simulink library "Renault_Library"  (Simulink 8.2)
settings: Solver=ode3  StartTime=0.0  StopTime=10.0  FixedStep=0.020  RelTol=1e-3
undefined symbols (not defined in the file or in its folder): ABC, AssystemProposal, b, chkval, CoeffA_C, CoeffB_C, CoeffMean_C, ConfTime_C, DiagDelay_C, DividePercent, FailureConfActive_C, FallingEdgeDuration_C, HighLevelThresholdVoltage_C, HighLevelVoltage_C, HighLevelVoltageTunable_C, InitValue_C, InputVoltRangeMax_C, InputVoltRangeMin_C, LowActiveContact_C, LowLevelThresholdVoltage_C, LowLevelVoltage_C, MaxOperatingVoltage_C, MinOperatingVoltage_C, mV2V_conversion, OUT1, OutOfRangeHigh_C, OutOfRangeLow_C, PinSupplySaturationMax, PinSupplySaturationMin, Res1Value_C, Res2Value_C, Res3Value_C, RisingEdgeDuration_C, RSA_CANSignalFailMonitoring, SampleTime_C, service14Table, service19Table, service22Table, service24Table, service28Table, service2ETable, service2FTable, service31Table, service87Table, SizeXStateDID2FTable, SizeXTable14, SizeXTable19, SizeXTable22, SizeXTable24, SizeXTable28, SizeXTable2E, SizeXTable2F, SizeXTable31, SizeXTable87, SizeYStateDID2FTable, SizeYTable14, SizeYTable19, SizeYTable22, SizeYTable24, SizeYTable28 ... (+22)
undefined symbols (not defined in the file): BatteryVoltage_ActivationThreshold_C, FrameStatus_AbsentValue_C, FrameStatus_ConfAbsentValue_C, MemThreshold_C, NAMEOFTHEMODEL_SAMPLE_TIME_CST, NbAbs1_C, NbConfAbs1_C, NbConfPres1_C, NbPres1_C, NOMDUSIGNAL_FaultConfTime_C, NOMDUSIGNAL_FaultRehabTime_C, NOMDUSIGNAL_switchvalue_C, NOMDUSIGNAL_unavailablevalue_C, PeriodFrame1_C, ResetTime_C, WakeUpTime_C
libraries: Renault_Library x112, simulink x75
(part 16 of 32)

== system Renault_Library/Complex blocks/Signal Routing/RSA_SlopeSwitch2Inputs/Slope_On_Block/Output_Comparision ==
  Test_passed : Inport
      settings: AttributesFormatString=Type : %<OutDataTypeStr>
  Input : Inport  Tag=AM,In,0,1,Name,C;CMP,Port,PortWidth,SampleTime;  Port=2
      settings: AttributesFormatString=Type : %<OutDataTypeStr>
  Pos_Slope : Inport  Tag=AM,In,0,1,Name,C;CMP,Port,PortWidth,SampleTime;  Port=3
      settings: AttributesFormatString=Type : %<OutDataTypeStr>
  Prev_Output : Inport  Port=4
      settings: AttributesFormatString=Type : %<OutDataTypeStr>
  Neg_Slope : Inport  Tag=AM,In,0,1,Name,C;CMP,Port,PortWidth,SampleTime;  Port=5
      settings: AttributesFormatString=Type : %<OutDataTypeStr>
  Logical_Operator : Logic  Operator=OR  OutDataTypeStr=boolean
  Logical_Operator2 : Logic  OutDataTypeStr=boolean
  Relational_Operator1 : RelationalOperator  Operator=>  OutDataTypeStr=boolean  InputSameDT=on
  Relational_Operator2 : RelationalOperator  Operator=<  OutDataTypeStr=boolean  InputSameDT=on
  Sum : Sum  OutDataTypeStr=Inherit: Inherit via back propagation  RndMeth=Zero  SaturateOnIntegerOverflow=on  InputSameDT=on
      settings: AttributesFormatString=SAT = %<SaturateOnIntegerOverflow>
  Sum1 : Sum  OutDataTypeStr=Inherit: Inherit via back propagation  RndMeth=Zero  SaturateOnIntegerOverflow=on  InputSameDT=on
      settings: AttributesFormatString=SAT = %<SaturateOnIntegerOverflow>
  Switch1 : Switch  Tag=GEN,Threshold;AM,Switch,2,1;CMP,Threshold;  Criteria=u2 ~= 0  RndMeth=Zero  SaturateOnIntegerOverflow=on  InputSameDT=on
      settings: AttributesFormatString=Criteria=%<Criteria>
  Switch2 : Switch  Tag=GEN,Threshold;AM,Switch,2,1;CMP,Threshold;  Criteria=u2 ~= 0  RndMeth=Zero  SaturateOnIntegerOverflow=on  InputSameDT=on
      settings: AttributesFormatString=Criteria=%<Criteria>
  Switch3 : Switch  Tag=GEN,Threshold;AM,Switch,2,1;CMP,Threshold;  Criteria=u2 ~= 0  RndMeth=Zero  SaturateOnIntegerOverflow=on  InputSameDT=on
      settings: AttributesFormatString=Criteria=%<Criteria>
  Output_Not_Equals_Input : Outport  Tag=AM,Out,1,0,Name,NC;CMP,Port,OutputWhenDisabled,InitialOutput;
      settings: AttributesFormatString=Type : %<OutDataTypeStr>\nInit :%<InitialOutput>
  Output : Outport  Tag=AM,Out,1,0,Name,NC;CMP,Port,OutputWhenDisabled,InitialOutput;  Port=2
      settings: AttributesFormatString=Type : %<OutDataTypeStr>\nInit :%<InitialOutput>
  RelOp2_out : Outport  Tag=AM,Out,1,0,Name,NC;CMP,Port,OutputWhenDisabled,InitialOutput;  Port=3
      settings: AttributesFormatString=Type : %<OutDataTypeStr>\nInit :%<InitialOutput>
  RelOp1_out : Outport  Tag=AM,Out,1,0,Name,NC;CMP,Port,OutputWhenDisabled,InitialOutput;  Port=4
      settings: AttributesFormatString=Type : %<OutDataTypeStr>\nInit :%<InitialOutput>
  wires:
  [RelOp1_out] -> RelOp1_out/1
  [RelOp2_out] -> RelOp2_out/1
  Relational_Operator2/1 -> [RelOp2_out]
  [RelOp1_out] -> Logical_Operator/2
  [Sum_out] -> Switch1/1
  [RelOp2_out] -> Logical_Operator/1
  [RelOp2_out] -> Switch2/2
  [Input] -> Switch3/3
  [Input] -> Switch1/3, Relational_Operator1/2
  [Input] -> Relational_Operator2/2
  Input/1 -> [Input]
  [sw2_out] -> Switch3/1
  Switch2/1 -> [sw2_out]
  [sw1_out] -> Switch2/3
  Switch1/1 -> [sw1_out]
  [Prev_Output] -> Sum/1
  Logical_Operator2/1 -> Output_Not_Equals_Input/1
  Logical_Operator/1 -> Logical_Operator2/2
  Switch3/1 -> Output/1
  Test_passed/1 -> Switch3/2, Logical_Operator2/1
  Relational_Operator1/1 -> [RelOp1_out], Switch1/2
  Sum/1 -> Relational_Operator1/1, [Sum_out]
  Sum1/1 -> Switch2/1, Relational_Operator2/1
  Pos_Slope/1 -> Sum1/2
  Prev_Output/1 -> Sum1/1, [Prev_Output]
  Neg_Slope/1 -> Sum/2

== system Renault_Library/Services ECU/Commonly_Used_Blocks/RSA_ServECU_EnabledSupply/Mean/Moy_1 ==
  In1 : Inport
  CoeffB2 : Constant  OutDataTypeStr=Inherit: Inherit via back propagation  SampleTime=-1
  Divide1 : Product  Inputs=*/  RndMeth=Zero  InputSameDT=on
  Out1 : Outport
  wires:
  CoeffB2/1 -> Divide1/2
  In1/1 -> Divide1/1
  Divide1/1 -> Out1/1

== system Renault_Library/Services ECU/Commonly_Used_Blocks/RSA_ServECU_EnabledSupply/Mean/Moy_10 ==
  In1 : Inport
  CoeffB2 : Constant  Value=10  OutDataTypeStr=Inherit: Inherit via back propagation  SampleTime=-1
  Divide1 : Product  Inputs=*/  RndMeth=Zero  InputSameDT=on
  Output{1..9} (9 alike) : UnitDelay  SampleTime=-1
  SumofElements2 : Sum  Inputs=10  CollapseDim=10  RndMeth=Zero  InputSameDT=on
  Out1 : Outport
  wires:
  SumofElements2/1 -> Divide1/1
  Output8/1 -> SumofElements2/9, Output9/1
  Output9/1 -> SumofElements2/10
  Output7/1 -> SumofElements2/8, Output8/1
  Output6/1 -> SumofElements2/7, Output7/1
  Output5/1 -> SumofElements2/6, Output6/1
  Output4/1 -> SumofElements2/5, Output5/1
  Output3/1 -> SumofElements2/4, Output4/1
  CoeffB2/1 -> Divide1/2
  Output2/1 -> Output3/1, SumofElements2/3
  Output1/1 -> Output2/1, SumofElements2/2
  In1/1 -> Output1/1, SumofElements2/1
  Divide1/1 -> Out1/1

== system Renault_Library/Services ECU/Commonly_Used_Blocks/RSA_ServECU_EnabledSupply/Mean/Moy_2 ==
  In1 : Inport
  CoeffB2 : Constant  Value=2  OutDataTypeStr=Inherit: Inherit via back propagation  SampleTime=-1
  Divide1 : Product  Inputs=*/  RndMeth=Zero  InputSameDT=on
  Output1 : UnitDelay  SampleTime=-1
  SumofElements2 : Sum  Inputs=2  CollapseDim=10  RndMeth=Zero  InputSameDT=on
  Out1 : Outport
  wires:
  SumofElements2/1 -> Divide1/1
  CoeffB2/1 -> Divide1/2
  Output1/1 -> SumofElements2/2
  In1/1 -> Output1/1, SumofElements2/1
  Divide1/1 -> Out1/1

== system Renault_Library/Services ECU/Commonly_Used_Blocks/RSA_ServECU_EnabledSupply/Mean/Moy_3 ==
  In1 : Inport
  CoeffB2 : Constant  Value=3  OutDataTypeStr=Inherit: Inherit via back propagation  SampleTime=-1
  Divide1 : Product  Inputs=*/  RndMeth=Zero  InputSameDT=on
  Output1 : UnitDelay  SampleTime=-1
  Output2 : UnitDelay  SampleTime=-1
  SumofElements1 : Sum  Inputs=2  CollapseDim=10  RndMeth=Zero  InputSameDT=on
  SumofElements2 : Sum  Inputs=2  CollapseDim=10  RndMeth=Zero  InputSameDT=on
  Out1 : Outport
  wires:
  SumofElements2/1 -> Divide1/1
  CoeffB2/1 -> Divide1/2
  Output2/1 -> SumofElements2/2
  Output1/1 -> Output2/1, SumofElements1/2
  In1/1 -> Output1/1, SumofElements1/1
  Divide1/1 -> Out1/1
  SumofElements1/1 -> SumofElements2/1

== system Renault_Library/Services ECU/Commonly_Used_Blocks/RSA_ServECU_EnabledSupply/Mean/Moy_4 ==
  In1 : Inport
  CoeffB2 : Constant  Value=4  OutDataTypeStr=Inherit: Inherit via back propagation  SampleTime=-1
  Divide1 : Product  Inputs=*/  RndMeth=Zero  InputSameDT=on
  Output1 : UnitDelay  SampleTime=-1
  Output2 : UnitDelay  SampleTime=-1
  Output3 : UnitDelay  SampleTime=-1
  SumofElements1 : Sum  Inputs=2  CollapseDim=10  RndMeth=Zero  InputSameDT=on
  SumofElements2 : Sum  Inputs=2  CollapseDim=10  RndMeth=Zero  InputSameDT=on
  SumofElements3 : Sum  Inputs=2  CollapseDim=10  RndMeth=Zero  InputSameDT=on
  Out1 : Outport
  wires:
  SumofElements3/1 -> Divide1/1
  Output3/1 -> SumofElements3/2
  CoeffB2/1 -> Divide1/2
  Output2/1 -> Output3/1, SumofElements2/2
  Output1/1 -> Output2/1, SumofElements1/2
  In1/1 -> Output1/1, SumofElements1/1
  Divide1/1 -> Out1/1
  SumofElements1/1 -> SumofElements2/1
  SumofElements2/1 -> SumofElements3/1

== system Renault_Library/Services ECU/Commonly_Used_Blocks/RSA_ServECU_EnabledSupply/Mean/Moy_5 ==
  In1 : Inport
  CoeffB2 : Constant  Value=5  OutDataTypeStr=Inherit: Inherit via back propagation  SampleTime=-1
  Divide1 : Product  Inputs=*/  RndMeth=Zero  InputSameDT=on
  Output{1..4} (4 alike) : UnitDelay  SampleTime=-1
  SumofElements{1..4} (4 alike) : Sum  Inputs=2  CollapseDim=10  RndMeth=Zero  InputSameDT=on
  Out1 : Outport
  wires:
  Output4/1 -> SumofElements4/2
  Output3/1 -> Output4/1, SumofElements3/2
  CoeffB2/1 -> Divide1/2
  Output2/1 -> Output3/1, SumofElements2/2
  Output1/1 -> Output2/1, SumofElements1/2
  In1/1 -> Output1/1, SumofElements1/1
  Divide1/1 -> Out1/1
  SumofElements3/1 -> SumofElements4/1
  SumofElements1/1 -> SumofElements2/1
  SumofElements2/1 -> SumofElements3/1
  SumofElements4/1 -> Divide1/1

== system Renault_Library/Services ECU/Commonly_Used_Blocks/RSA_ServECU_EnabledSupply/Mean/Moy_6 ==
  In1 : Inport
  CoeffB2 : Constant  Value=6  OutDataTypeStr=Inherit: Inherit via back propagation  SampleTime=-1
  Divide1 : Product  Inputs=*/  RndMeth=Zero  InputSameDT=on
  Output{1..5} (5 alike) : UnitDelay  SampleTime=-1
  SumofElements{1..5} (5 alike) : Sum  Inputs=2  CollapseDim=10  RndMeth=Zero  InputSameDT=on
  Out1 : Outport
  wires:
  SumofElements5/1 -> Divide1/1
  Output5/1 -> SumofElements5/2
  Output4/1 -> Output5/1, SumofElements4/2
  Output3/1 -> Output4/1, SumofElements3/2
  CoeffB2/1 -> Divide1/2
  Output2/1 -> Output3/1, SumofElements2/2
  Output1/1 -> Output2/1, SumofElements1/2
  In1/1 -> Output1/1, SumofElements1/1
  Divide1/1 -> Out1/1
  SumofElements1/1 -> SumofElements2/1
  SumofElements2/1 -> SumofElements3/1
  SumofElements3/1 -> SumofElements4/1
  SumofElements4/1 -> SumofElements5/1

== system Renault_Library/Services ECU/Commonly_Used_Blocks/RSA_ServECU_EnabledSupply/Mean/Moy_7 ==
  In1 : Inport
  CoeffB2 : Constant  Value=7  OutDataTypeStr=Inherit: Inherit via back propagation  SampleTime=-1
  Divide1 : Product  Inputs=*/  RndMeth=Zero  InputSameDT=on
  Output{1..6} (6 alike) : UnitDelay  SampleTime=-1
  SumofElements{1..6} (6 alike) : Sum  Inputs=2  CollapseDim=10  RndMeth=Zero  InputSameDT=on
  Out1 : Outport
  wires:
  SumofElements6/1 -> Divide1/1
  Output6/1 -> SumofElements6/2
  Output5/1 -> Output6/1, SumofElements5/2
  Output4/1 -> Output5/1, SumofElements4/2
  Output3/1 -> Output4/1, SumofElements3/2
  CoeffB2/1 -> Divide1/2
  Output2/1 -> Output3/1, SumofElements2/2
  Output1/1 -> Output2/1, SumofElements1/2
  In1/1 -> Output1/1, SumofElements1/1
  Divide1/1 -> Out1/1
  SumofElements1/1 -> SumofElements2/1
  SumofElements2/1 -> SumofElements3/1
  SumofElements3/1 -> SumofElements4/1
  SumofElements4/1 -> SumofElements5/1
  SumofElements5/1 -> SumofElements6/1

== system Renault_Library/Services ECU/Commonly_Used_Blocks/RSA_ServECU_EnabledSupply/Mean/Moy_8 ==
  In1 : Inport
  CoeffB2 : Constant  Value=8  OutDataTypeStr=Inherit: Inherit via back propagation  SampleTime=-1
  Divide1 : Product  Inputs=*/  RndMeth=Zero  InputSameDT=on
  Output{1..7} (7 alike) : UnitDelay  SampleTime=-1
  SumofElements{1..7} (7 alike) : Sum  Inputs=2  CollapseDim=10  RndMeth=Zero  InputSameDT=on
  Out1 : Outport
  wires:
  Output7/1 -> SumofElements7/2
  Output6/1 -> Output7/1, SumofElements6/2
  Output5/1 -> Output6/1, SumofElements5/2
  Output4/1 -> Output5/1, SumofElements4/2
  Output3/1 -> Output4/1, SumofElements3/2
  CoeffB2/1 -> Divide1/2
  Output2/1 -> Output3/1, SumofElements2/2
  Output1/1 -> Output2/1, SumofElements1/2
  In1/1 -> Output1/1, SumofElements1/1
  Divide1/1 -> Out1/1
  SumofElements7/1 -> Divide1/1
  SumofElements5/1 -> SumofElements6/1
  SumofElements4/1 -> SumofElements5/1
  SumofElements3/1 -> SumofElements4/1
  SumofElements2/1 -> SumofElements3/1
  SumofElements1/1 -> SumofElements2/1
  SumofElements6/1 -> SumofElements7/1

== system Renault_Library/Services ECU/Commonly_Used_Blocks/RSA_ServECU_EnabledSupply/Mean/Moy_9 ==
  In1 : Inport
  CoeffB2 : Constant  Value=9  OutDataTypeStr=Inherit: Inherit via back propagation  SampleTime=-1
  Divide1 : Product  Inputs=*/  RndMeth=Zero  InputSameDT=on
  Output{1..8} (8 alike) : UnitDelay  SampleTime=-1
  SumofElements2 : Sum  Inputs=9  CollapseDim=10  RndMeth=Zero  InputSameDT=on
  Out1 : Outport
  wires:
  SumofElements2/1 -> Divide1/1
  Output8/1 -> SumofElements2/9
  Output7/1 -> SumofElements2/8, Output8/1
  Output6/1 -> SumofElements2/7, Output7/1
  Output5/1 -> SumofElements2/6, Output6/1
  Output4/1 -> SumofElements2/5, Output5/1
  Output3/1 -> SumofElements2/4, Output4/1
  CoeffB2/1 -> Divide1/2
  Output2/1 -> Output3/1, SumofElements2/3
  Output1/1 -> Output2/1, SumofElements2/2
  In1/1 -> Output1/1, SumofElements2/1
  Divide1/1 -> Out1/1

== system Renault_Library/Services ECU/Diag/RSA_DiagnosticManagement/DIAGNOSTIC_SANCTION_TOP/GeneralService ==
  SEG_STATE : Inport
  DIAG_BUFFER_REQUEST : Inport  Port=2
  SESSION_ACTIVE : Inport  Port=3
  REQ_DATA_NUMBER : Inport  Port=4
  General_Server_Response : Stateflow chart  TreatAsAtomicUnit=on
  Server_State : Subsystem
  Service_Supported : Subsystem
  Service_Supported_In_Active_Session : Subsystem
  NEGATIVE_RES_CODE : Outport
  NUMBER_SERVICE : Outport  Port=2  OutDataTypeStr=uint8
  SEND_RES_MESSAGE : Outport  Port=3  OutDataTypeStr=boolean
  REQUEST_RECEIVED : Outport  Port=4  OutDataTypeStr=boolean
  wires:
  General_Server_Response/1(NEGATIVE_RES_CODE) -> NEGATIVE_RES_CODE/1
  SEG_STATE/1 -> Server_State/1(SEG_STATE)
  DIAG_BUFFER_REQUEST/1 -> Service_Supported/1(DIAG_BUFFER_REQUEST), Service_Supported_In_Active_Session/1(DIAG_BUFFER_REQUEST), General_Server_Response/2(DIAG_BUFFER_REQUEST)
  Service_Supported/1(SERVICE_SUPPORTED) -> General_Server_Response/3(SERVICE_SUPPORTED)
  SESSION_ACTIVE/1 -> Service_Supported_In_Active_Session/2(SESSION_ACTIVE)
  Server_State/1(ECU_AVAILABLE) -> General_Server_Response/5(ECU_AVAILABLE)
  Service_Supported_In_Active_Session/1(SERVICE_SUPPORTED_IN_ACTIVE_SESSION) -> General_Server_Response/4(SERVICE_SUPPORTED_IN_ACTIVE_SESSION)
  General_Server_Response/2(NUMBER_SERVICE) -> NUMBER_SERVICE/1
  General_Server_Response/3(SEND_RES_MESSAGE) -> SEND_RES_MESSAGE/1
  General_Server_Response/4(REQUEST_RECEIVED) -> REQUEST_RECEIVED/1
  REQ_DATA_NUMBER/1 -> General_Server_Response/1(REQ_DATA_NUMBER)

== system Renault_Library/Services ECU/Diag/RSA_DiagnosticManagement/DIAGNOSTIC_SANCTION_TOP/Service10 ==
  DIAG_BUFFER_REQUEST : Inport
  REQ_DATA_NUMBER : Inport  Port=2
  Trigger : TriggerPort
  Check_Lenght_Message_Service10 : Subsystem
  Check_Message_Service10 : Stateflow chart  TreatAsAtomicUnit=on
  Constant : Constant  Value=true  OutDataTypeStr=boolean  SampleTime=-1
  SubFunction_Supported_Service10 : Subsystem
  SUPPRESS_POS_RES : Outport
  NEGATIVE_RES_CODE : Outport  Port=2
  SEND_RES_MESSAGE : Outport  Port=3
  wires:
  REQ_DATA_NUMBER/1 -> Check_Lenght_Message_Service10/1(REQ_DATA_NUMBER)
  SubFunction_Supported_Service10/2(SUBFUNCTION_SUPPORTED) -> Check_Message_Service10/1(SUBFUNCTION_SUPPORTED)
  Check_Lenght_Message_Service10/1(LENGTH_MESSAGE_CORRECT) -> Check_Message_Service10/2(LENGTH_MESSAGE_CORRECT)
  DIAG_BUFFER_REQUEST/1 -> SubFunction_Supported_Service10/1(DIAG_BUFFER_REQUEST)
  SubFunction_Supported_Service10/1(SUPPRESS_POS_RES) -> SUPPRESS_POS_RES/1
  Check_Message_Service10/1(NEGATIVE_RES_CODE) -> NEGATIVE_RES_CODE/1
  Constant/1 -> Check_Message_Service10/3(CONDITION_CORRECT)
  Check_Message_Service10/2(SEND_RES_MESSAGE) -> SEND_RES_MESSAGE/1

== system Renault_Library/Services ECU/Diag/RSA_DiagnosticManagement/DIAGNOSTIC_SANCTION_TOP/Service11 ==
  DIAG_BUFFER_REQUEST : Inport
  REQ_DATA_NUMBER : Inport  Port=2
  SESSION_ACTIVE : Inport  Port=3
  Trigger : TriggerPort
  Check_Lenght_Message_Service11 : Subsystem
  Check_Message_Service11 : Stateflow chart  TreatAsAtomicUnit=on
  Constant : Constant  Value=true  OutDataTypeStr=boolean  SampleTime=-1
  Constant1 : Constant  Value=true  OutDataTypeStr=boolean  SampleTime=-1
  SubFunction_In_Active_Session_Service11 : Subsystem
  SubFunction_Supported_Service11 : Subsystem
  SUPPRESS_POS_RES : Outport
  NEGATIVE_RES_CODE : Outport  Port=2
  SEND_RES_MESSAGE : Outport  Port=3
  wires:
  SESSION_ACTIVE/1 -> SubFunction_In_Active_Session_Service11/2(SESSION)
  Check_Message_Service11/1(NEGATIVE_RES_CODE) -> NEGATIVE_RES_CODE/1
  SubFunction_Supported_Service11/1(SUPPRESS_POS_RES) -> SUPPRESS_POS_RES/1
  Check_Lenght_Message_Service11/1(LENGTH_MESSAGE_CORRECT) -> Check_Message_Service11/3(LENGTH_MESSAGE_CORRECT)
  SubFunction_In_Active_Session_Service11/1(SUBFUNCTION_SUPPORTED_IN_ACTIVE_SESSION) -> Check_Message_Service11/2(SUBFUNCTION_SUPPORTED_IN_ACTIVE_SESSION)
  SubFunction_Supported_Service11/2(SUBFUNCTION_SUPPORTED) -> Check_Message_Service11/1(SUBFUNCTION_SUPPORTED)
  REQ_DATA_NUMBER/1 -> Check_Lenght_Message_Service11/1(REQ_DATA_NUMBER)
  DIAG_BUFFER_REQUEST/1 -> SubFunction_In_Active_Session_Service11/1(DIAG_BUFFER_REQUEST), SubFunction_Supported_Service11/1(DIAG_BUFFER_REQUEST)
  Check_Message_Service11/2(SEND_RES_MESSAGE) -> SEND_RES_MESSAGE/1
  Constant/1 -> Check_Message_Service11/4(CONDITION_CORRECT)
  Constant1/1 -> Check_Message_Service11/5(SECURITY_ACCESS_ACCEPTED)

== system Renault_Library/Services ECU/Diag/RSA_DiagnosticManagement/DIAGNOSTIC_SANCTION_TOP/Service14 ==
  REQ_DATA_NUMBER : Inport
  SESSION_ACTIVE : Inport  Port=2
  DIAG_BUFFER_REQUEST : Inport  Port=3
  Trigger : TriggerPort
  Check_Lenght_Message_Service14 : Subsystem
  Check_Message_Service14 : Stateflow chart  TreatAsAtomicUnit=on
  Constant : Constant  Value=true  OutDataTypeStr=boolean  SampleTime=-1
  Parameter_Valid_Service14 : Subsystem
  NEGATIVE_RES_CODE : Outport
  SEND_RES_MESSAGE : Outport  Port=2
  wires:
  REQ_DATA_NUMBER/1 -> Check_Lenght_Message_Service14/1(REQ_DATA_NUMBER)
  Check_Lenght_Message_Service14/1(LENGTH_MESSAGE_CORRECT) -> Check_Message_Service14/1(LENGTH_MESSAGE_CORRECT)
  Check_Message_Service14/1(NEGATIVE_RES_CODE) -> NEGATIVE_RES_CODE/1
  Check_Message_Service14/2(SEND_RES_MESSAGE) -> SEND_RES_MESSAGE/1
  SESSION_ACTIVE/1 -> Parameter_Valid_Service14/2(SESSION_ACTIVE)
  Parameter_Valid_Service14/1(PARAMETER_VALID) -> Check_Message_Service14/3(PARAMETER_VALID)
  DIAG_BUFFER_REQUEST/1 -> Parameter_Valid_Service14/1(DIAG_BUFFER_REQUEST)
  Constant/1 -> Check_Message_Service14/2(CONDITION_CORRECT)

== system Renault_Library/Services ECU/Diag/RSA_DiagnosticManagement/DIAGNOSTIC_SANCTION_TOP/Service19 ==
  REQ_DATA_NUMBER : Inport
  DIAG_BUFFER_REQUEST : Inport  Port=2
  SESSION_ACTIVE : Inport  Port=3
  Trigger : TriggerPort
  Check_Lenght_Message_Service19 : Subsystem
  Check_Message_Service19 : Stateflow chart  TreatAsAtomicUnit=on
  Parameter_Valid_Service19 : Subsystem
  SubFunction_In_Active_Session_Service19 : Subsystem
  SubFunction_Supported_Service19 : Subsystem
  NEGATIVE_RES_CODE : Outport
  SUPPRESS_POS_RES : Outport  Port=2
  SEND_RES_MESSAGE : Outport  Port=3
  wires:
  REQ_DATA_NUMBER/1 -> Check_Lenght_Message_Service19/1(REQ_DATA_NUMBER)
  Check_Lenght_Message_Service19/1(LENGTH_MESSAGE_CORRECT) -> Check_Message_Service19/1(LENGTH_MESSAGE_CORRECT)
  DIAG_BUFFER_REQUEST/1 -> SubFunction_In_Active_Session_Service19/2(DIAG_BUFFER_REQUEST), Parameter_Valid_Service19/1(DIAG_BUFFER_REQUEST), SubFunction_Supported_Service19/1(DIAG_BUFFER_REQUEST)
  SubFunction_Supported_Service19/1(SUBFUNCTION_SUPPORTED) -> Check_Message_Service19/4(SUBFUNCTION_SUPPORTED)
  SubFunction_Supported_Service19/2(SUPPRESS_POS_RES) -> SUPPRESS_POS_RES/1
  SESSION_ACTIVE/1 -> SubFunction_In_Active_Session_Service19/1(SESSION)
  SubFunction_In_Active_Session_Service19/1(SUBFUNCTION_SUPPORTED_IN_ACTIVE_SESSION) -> Check_Message_Service19/2(SUBFUNCTION_SUPPORTED_IN_ACTIVE_SESSION)
  Parameter_Valid_Service19/1(PARAMETER_VALID) -> Check_Message_Service19/3(PARAMETER_VALID)
  Check_Message_Service19/1(NEGATIVE_RES_CODE) -> NEGATIVE_RES_CODE/1
  Check_Message_Service19/2(SEND_RES_MESSAGE) -> SEND_RES_MESSAGE/1

== system Renault_Library/Services ECU/Diag/RSA_DiagnosticManagement/DIAGNOSTIC_SANCTION_TOP/Service22 ==
  REQ_DATA_NUMBER : Inport
  DIAG_BUFFER_REQUEST : Inport  Port=2
  SESSION_ACTIVE : Inport  Port=3
  Trigger : TriggerPort
  Check_Lenght_Message_Response_Service22 : Subsystem
  Check_Lenght_Message_Service22 : Subsystem
  Check_Message_Service22 : Stateflow chart  TreatAsAtomicUnit=on
  Constant : Constant  Value=true  OutDataTypeStr=boolean  SampleTime=-1
  Parameter_Valid_Service22 : Subsystem
  NEGATIVE_RES_CODE : Outport
  LIST_DID_SERVICE_22 : Outport  Port=2  PortDimensions=[2046 3]
  SEND_RES_MESSAGE : Outport  Port=3
  wires:
  Check_Lenght_Message_Service22/1(LENGTH_MESSAGE_CORRECT) -> Check_Message_Service22/1(LENGTH_MESSAGE_CORRECT)
  REQ_DATA_NUMBER/1 -> Check_Lenght_Message_Service22/1(REQ_DATA_NUMBER), Parameter_Valid_Service22/1(REQ_DATA_NUMBER)
  Check_Message_Service22/1(NEGATIVE_RES_CODE) -> NEGATIVE_RES_CODE/1
  DIAG_BUFFER_REQUEST/1 -> Parameter_Valid_Service22/2(DIAG_BUFFER_REQUEST)
  Parameter_Valid_Service22/3(PARAMETER_VALID) -> Check_Message_Service22/5(PARAMETER_VALID)
  Parameter_Valid_Service22/4(LIST_DID_SERVICE_22) -> LIST_DID_SERVICE_22/1
  Constant/1 -> Check_Message_Service22/2(CONDITION_CORRECT)
  Check_Lenght_Message_Response_Service22/1(LENGTH_RESPONSE_CORRECT) -> Check_Message_Service22/3(LENGTH_RESPONSE_CORRECT)
  Parameter_Valid_Service22/1(RESPONSE_SIZE) -> Check_Lenght_Message_Response_Service22/1(RESPONSE_SIZE)
  Parameter_Valid_Service22/2(SECURITY_ACCESS_ACCEPTED) -> Check_Message_Service22/4(SECURITY_ACCESS_ACCEPTED)
  SESSION_ACTIVE/1 -> Parameter_Valid_Service22/3(SESSION_ACTIVE)
  Check_Message_Service22/2(SEND_RES_MESSAGE) -> SEND_RES_MESSAGE/1

== system Renault_Library/Services ECU/Diag/RSA_DiagnosticManagement/DIAGNOSTIC_SANCTION_TOP/Service23 ==
  REQ_DATA_NUMBER : Inport
  DIAG_BUFFER_REQUEST : Inport  Port=2
  SESSION_ACTIVE : Inport  Port=3
  Trigger : TriggerPort
  Check_Lenght_Message_Service23 : Subsystem
  Check_Message_Service23 : Stateflow chart  TreatAsAtomicUnit=on
  Constant : Constant  Value=true  OutDataTypeStr=boolean  SampleTime=-1
  Parameter_Valid_Service23 : Subsystem
  NEGATIVE_RES_CODE : Outport
  SEND_RES_MESSAGE : Outport  Port=2
  wires:
  Check_Lenght_Message_Service23/1(LENGTH_MESSAGE_CORRECT) -> Check_Message_Service23/1(LENGTH_MESSAGE_CORRECT)
  REQ_DATA_NUMBER/1 -> Check_Lenght_Message_Service23/1(REQ_DATA_NUMBER), Parameter_Valid_Service23/1(REQ_DATA_NUMBER)
  Check_Message_Service23/1(NEGATIVE_RES_CODE) -> NEGATIVE_RES_CODE/1
  DIAG_BUFFER_REQUEST/1 -> Parameter_Valid_Service23/2(DIAG_BUFFER_REQUEST)
  Constant/1 -> Check_Message_Service23/2(CONDITION_CORRECT)
  SESSION_ACTIVE/1 -> Parameter_Valid_Service23/3(SESSION_ACTIVE)
  Check_Message_Service23/2(SEND_RES_MESSAGE) -> SEND_RES_MESSAGE/1
  Parameter_Valid_Service23/1(SECURITY_ACCESS_ACCEPTED) -> Check_Message_Service23/3(SECURITY_ACCESS_ACCEPTED)
  Parameter_Valid_Service23/2(PARAMETER_VALID) -> Check_Message_Service23/4(PARAMETER_VALID)

== system Renault_Library/Services ECU/Diag/RSA_DiagnosticManagement/DIAGNOSTIC_SANCTION_TOP/Service24 ==
  REQ_DATA_NUMBER : Inport
  DIAG_BUFFER_REQUEST : Inport  Port=2
  SESSION_ACTIVE : Inport  Port=3
  Trigger : TriggerPort
  Check_Lenght_Message_Service24 : Subsystem
  Check_Message_Service24 : Stateflow chart  TreatAsAtomicUnit=on
  Constant : Constant  Value=true  OutDataTypeStr=boolean  SampleTime=-1
  Parameter_Valid_Service24 : Subsystem
  NEGATIVE_RES_CODE : Outport
  SEND_RES_MESSAGE : Outport  Port=2
  wires:
  Check_Lenght_Message_Service24/1(LENGTH_MESSAGE_CORRECT) -> Check_Message_Service24/1(LENGTH_MESSAGE_CORRECT)
  REQ_DATA_NUMBER/1 -> Check_Lenght_Message_Service24/1(REQ_DATA_NUMBER)
  Check_Message_Service24/1(NEGATIVE_RES_CODE) -> NEGATIVE_RES_CODE/1
  DIAG_BUFFER_REQUEST/1 -> Parameter_Valid_Service24/1(DIAG_BUFFER_REQUEST)
  Parameter_Valid_Service24/2(PARAMETER_VALID) -> Check_Message_Service24/4(PARAMETER_VALID)
  Constant/1 -> Check_Message_Service24/2(CONDITION_CORRECT)
  Parameter_Valid_Service24/1(SECURITY_ACCESS_ACCEPTED) -> Check_Message_Service24/3(SECURITY_ACCESS_ACCEPTED)
  SESSION_ACTIVE/1 -> Parameter_Valid_Service24/2(SESSION_ACTIVE)
  Check_Message_Service24/2(SEND_RES_MESSAGE) -> SEND_RES_MESSAGE/1

== system Renault_Library/Services ECU/Diag/RSA_DiagnosticManagement/DIAGNOSTIC_SANCTION_TOP/Service27 ==
  REQ_DATA_NUMBER : Inport
  DIAG_BUFFER_REQUEST : Inport  Port=2
  SESSION_ACTIVE : Inport  Port=3
  Trigger : TriggerPort
  Check_Lenght_Message_Service27 : Subsystem
  Check_Message_Service27 : Stateflow chart  TreatAsAtomicUnit=on
  Constant{,1..3} (4 alike) : Constant  Value=true  OutDataTypeStr=boolean  SampleTime=-1
  SubFunction_In_Active_Session_Service27 : Subsystem
  SubFunction_Supported_Service27 : Subsystem
  NEGATIVE_RES_CODE : Outport
  SUPPRESS_POS_RES : Outport  Port=2
  SEND_RES_MESSAGE : Outport  Port=3
  wires:
  REQ_DATA_NUMBER/1 -> Check_Lenght_Message_Service27/1(REQ_DATA_NUMBER)
  Check_Lenght_Message_Service27/1(LENGTH_MESSAGE_CORRECT) -> Check_Message_Service27/1(LENGTH_MESSAGE_CORRECT)
  DIAG_BUFFER_REQUEST/1 -> SubFunction_Supported_Service27/1(DIAG_BUFFER_REQUEST), SubFunction_In_Active_Session_Service27/2(DIAG_BUFFER_REQUEST)
  SubFunction_Supported_Service27/1(SUBFUNCTION_SUPPORTED) -> Check_Message_Service27/7(SUBFUNCTION_SUPPORTED)
  SubFunction_Supported_Service27/2(SUPPRESS_POS_RES) -> SUPPRESS_POS_RES/1
  Check_Message_Service27/1(NEGATIVE_RES_CODE) -> NEGATIVE_RES_CODE/1
  SESSION_ACTIVE/1 -> SubFunction_In_Active_Session_Service27/1(SESSION)
  SubFunction_In_Active_Session_Service27/1(SUBFUNCTION_SUPPORTED_IN_ACTIVE_SESSION) -> Check_Message_Service27/6(SUBFUNCTION_SUPPORTED_IN_ACTIVE_SESSION)
  Check_Message_Service27/2(SEND_RES_MESSAGE) -> SEND_RES_MESSAGE/1
  Constant/1 -> Check_Message_Service27/2(CONDITION_CORRECT)
  Constant1/1 -> Check_Message_Service27/3(REQ_SEQUENCE_OK)
  Constant2/1 -> Check_Message_Service27/4(KEY_VALID)
  Constant3/1 -> Check_Message_Service27/5(PARAMETER_VALID)

== system Renault_Library/Services ECU/Diag/RSA_DiagnosticManagement/DIAGNOSTIC_SANCTION_TOP/Service28 ==
  REQ_DATA_NUMBER : Inport
  DIAG_BUFFER_REQUEST : Inport  Port=2
  SESSION_ACTIVE : Inport  Port=3
  Trigger : TriggerPort
  Check_Lenght_Message_Service28 : Subsystem
  Check_Message_Service28 : Stateflow chart  TreatAsAtomicUnit=on
  Constant : Constant  Value=true  OutDataTypeStr=boolean  SampleTime=-1
  Parameter_Valid_Service28 : Subsystem
  SubFunction_In_Active_Session_Service28 : Subsystem
  SubFunction_Supported_Service28 : Subsystem
  NEGATIVE_RES_CODE : Outport
  SUPPRESS_POS_RES : Outport  Port=2
  SEND_RES_MESSAGE : Outport  Port=3
  wires:
  REQ_DATA_NUMBER/1 -> Check_Lenght_Message_Service28/1(REQ_DATA_NUMBER)
  DIAG_BUFFER_REQUEST/1 -> SubFunction_Supported_Service28/1(DIAG_BUFFER_OUTPUT), SubFunction_In_Active_Session_Service28/2(DIAG_BUFFER_REQUEST), Parameter_Valid_Service28/1(DIAG_BUFFER_REQUEST)
  SubFunction_Supported_Service28/1(SUBFUNCTION_SUPPORTED) -> Check_Message_Service28/5(SUBFUNCTION_SUPPORTED)
  Check_Message_Service28/1(NEGATIVE_RES_CODE) -> NEGATIVE_RES_CODE/1
  SubFunction_Supported_Service28/2(SUPPRESS_POS_RES) -> SUPPRESS_POS_RES/1
  SubFunction_In_Active_Session_Service28/1(SUBFUNCTION_SUPPORTED_IN_ACTIVE_SESSION) -> Check_Message_Service28/3(SUBFUNCTION_SUPPORTED_IN_ACTIVE_SESSION)
  SESSION_ACTIVE/1 -> SubFunction_In_Active_Session_Service28/1(SESSION)
  Check_Lenght_Message_Service28/1(LENGTH_MESSAGE_CORRECT) -> Check_Message_Service28/1(LENGTH_MESSAGE_CORRECT)
  Check_Message_Service28/2(SEND_RES_MESSAGE) -> SEND_RES_MESSAGE/1
  Parameter_Valid_Service28/1(PARAMETER_VALID) -> Check_Message_Service28/4(PARAMETER_VALID)
  Constant/1 -> Check_Message_Service28/2(CONDITION_CORRECT)

== system Renault_Library/Services ECU/Diag/RSA_DiagnosticManagement/DIAGNOSTIC_SANCTION_TOP/Service2E ==
  REQ_DATA_NUMBER : Inport
  DIAG_BUFFER_REQUEST : Inport  Port=2
  SESSION_ACTIVE : Inport  Port=3
  Trigger : TriggerPort
  Check_Lenght_Message_Service2E : Subsystem
  Check_Message_Service2E : Stateflow chart  TreatAsAtomicUnit=on
  Constant : Constant  Value=true  OutDataTypeStr=boolean  SampleTime=-1
  Constant1 : Constant  Value=true  OutDataTypeStr=boolean  SampleTime=-1
  Parameter_Valid_Service2E : Subsystem
  NEGATIVE_RES_CODE : Outport
  SEND_RES_MESSAGE : Outport  Port=2
  wires:
  REQ_DATA_NUMBER/1 -> Check_Lenght_Message_Service2E/1(REQ_DATA_NUMBER), Parameter_Valid_Service2E/1(REQ_DATA_NUMBER)
  Check_Lenght_Message_Service2E/1(LENGTH_MESSAGE_CORRECT) -> Check_Message_Service2E/1(LENGTH_MESSAGE_CORRECT)
  Constant/1 -> Check_Message_Service2E/2(CONDITION_CORRECT)
  Constant1/1 -> Check_Message_Service2E/3(CORRECTLY_ALTERED_INTO_MEMORY)
  DIAG_BUFFER_REQUEST/1 -> Parameter_Valid_Service2E/3(DIAG_BUFFER_REQUEST)
  SESSION_ACTIVE/1 -> Parameter_Valid_Service2E/2(SESSION_ACTIVE)
  Parameter_Valid_Service2E/2(PARAMETER_VALID) -> Check_Message_Service2E/5(PARAMETER_VALID)
  Parameter_Valid_Service2E/1(SECURITY_ACCESS_ACCEPTED) -> Check_Message_Service2E/4(SECURITY_ACCESS_ACCEPTED)
  Check_Message_Service2E/2(SEND_RES_MESSAGE) -> SEND_RES_MESSAGE/1
  Check_Message_Service2E/1(NEGATIVE_RES_CODE) -> NEGATIVE_RES_CODE/1

== system Renault_Library/Services ECU/Diag/RSA_DiagnosticManagement/DIAGNOSTIC_SANCTION_TOP/Service2F ==
  REQ_DATA_NUMBER : Inport
  SESSION_ACTIVE : Inport  Port=2
  DIAG_BUFFER_REQUEST : Inport  Port=3
  Trigger : TriggerPort
  Check_Lenght_Message_Service2F : Subsystem
  Check_Message_Service2F : Stateflow chart  TreatAsAtomicUnit=on
  Constant : Constant  Value=true  OutDataTypeStr=boolean  SampleTime=-1
  Parameter_Valid_Service2F : Subsystem
  NEGATIVE_RES_CODE : Outport
  SEND_RES_MESSAGE : Outport  Port=2
  wires:
  REQ_DATA_NUMBER/1 -> Check_Lenght_Message_Service2F/1(REQ_DATA_NUMBER)
  Check_Lenght_Message_Service2F/1(LENGTH_MESSAGE_CORRECT) -> Check_Message_Service2F/1(LENGTH_MESSAGE_CORRECT)
  Check_Message_Service2F/1(NEGATIVE_RES_CODE) -> NEGATIVE_RES_CODE/1
  Check_Message_Service2F/2(SEND_RES_MESSAGE) -> SEND_RES_MESSAGE/1
  Parameter_Valid_Service2F/2(PARAMETER_VALID) -> Check_Message_Service2F/4(PARAMETER_VALID)
  SESSION_ACTIVE/1 -> Parameter_Valid_Service2F/1(SESSION_ACTIVE)
  DIAG_BUFFER_REQUEST/1 -> Parameter_Valid_Service2F/2(DIAG_BUFFER_REQUEST)
  Parameter_Valid_Service2F/1(SECURITY_ACCESS_ACCEPTED) -> Check_Message_Service2F/3(SECURITY_ACCESS_ACCEPTED)
  Constant/1 -> Check_Message_Service2F/2(CONDITION_CORRECT)

== system Renault_Library/Services ECU/Diag/RSA_DiagnosticManagement/DIAGNOSTIC_SANCTION_TOP/Service31 ==
  REQ_DATA_NUMBER : Inport
  DIAG_BUFFER_REQUEST : Inport  Port=2
  SESSION_ACTIVE : Inport  Port=3
  Trigger : TriggerPort
  Check_Lenght_Message_Service31 : Subsystem
  Check_Message_Service31 : Stateflow chart  TreatAsAtomicUnit=on
  Constant : Constant  Value=true  OutDataTypeStr=boolean  SampleTime=-1
  Constant1 : Constant  Value=true  OutDataTypeStr=boolean  SampleTime=-1
  Parameter_Valid_Service31 : Subsystem
  SubFunction_In_Active_Session_Service31 : Subsystem
  SubFunction_Supported_Service31 : Subsystem
  NEGATIVE_RES_CODE : Outport
  SUPPRESS_POS_RES : Outport  Port=2
  SEND_RES_MESSAGE : Outport  Port=3
  wires:
  REQ_DATA_NUMBER/1 -> Check_Lenght_Message_Service31/1(REQ_DATA_NUMBER)
  Check_Lenght_Message_Service31/1(LENGTH_MESSAGE_CORRECT) -> Check_Message_Service31/1(LENGTH_MESSAGE_CORRECT)
  Check_Message_Service31/1(NEGATIVE_RES_CODE) -> NEGATIVE_RES_CODE/1
  SubFunction_Supported_Service31/1(SUBFUNCTION_SUPPORTED) -> Check_Message_Service31/7(SUBFUNCTION_SUPPORTED)
  DIAG_BUFFER_REQUEST/1 -> SubFunction_In_Active_Session_Service31/2(DIAG_BUFFER_REQUEST), SubFunction_Supported_Service31/1(DIAG_BUFFER_REQUEST), [DIAG_BUFFER_REQUEST]
  SubFunction_Supported_Service31/2(SUPPRESS_POS_RES) -> SUPPRESS_POS_RES/1
  SubFunction_In_Active_Session_Service31/1(SUBFUNCTION_SUPPORTED_IN_ACTIVE_SESSION) -> Check_Message_Service31/6(SUBFUNCTION_SUPPORTED_IN_ACTIVE_SESSION)
  SESSION_ACTIVE/1 -> SubFunction_In_Active_Session_Service31/1(SESSION), Parameter_Valid_Service31/2(SESSION_ACTIVE)
  Check_Message_Service31/2(SEND_RES_MESSAGE) -> SEND_RES_MESSAGE/1
  [DIAG_BUFFER_REQUEST] -> Parameter_Valid_Service31/1(DIAG_BUFFER_REQUEST)
  Parameter_Valid_Service31/1(SECURITY_ACCESS_ACCEPTED) -> Check_Message_Service31/4(SECURITY_ACCESS_ACCEPTED)
  Parameter_Valid_Service31/2(PARAMETER_VALID) -> Check_Message_Service31/5(PARAMETER_VALID)
  Constant/1 -> Check_Message_Service31/2(REQ_SEQUENCE_OK)
  Constant1/1 -> Check_Message_Service31/3(CONDITION_CORRECT)

== system Renault_Library/Services ECU/Diag/RSA_DiagnosticManagement/DIAGNOSTIC_SANCTION_TOP/Service3D ==
  REQ_DATA_NUMBER : Inport
  DIAG_BUFFER_REQUEST : Inport  Port=2
  SESSION_ACTIVE : Inport  Port=3
  Trigger : TriggerPort
  Check_Lenght_Message_Service3D : Subsystem
  Check_Message_Service3D : Stateflow chart  TreatAsAtomicUnit=on
  Constant : Constant  Value=true  OutDataTypeStr=boolean  SampleTime=-1
  Constant1 : Constant  Value=true  OutDataTypeStr=boolean  SampleTime=-1
  Parameter_Valid_Service3D : Subsystem
  NEGATIVE_RES_CODE : Outport
  SEND_RES_MESSAGE : Outport  Port=2
  wires:
  Check_Message_Service3D/1(NEGATIVE_RES_CODE) -> NEGATIVE_RES_CODE/1
  Check_Message_Service3D/2(SEND_RES_MESSAGE) -> SEND_RES_MESSAGE/1
  SESSION_ACTIVE/1 -> Parameter_Valid_Service3D/2(SESSION_ACTIVE)
  DIAG_BUFFER_REQUEST/1 -> Parameter_Valid_Service3D/3(DIAG_BUFFER_REQUEST)
  Constant1/1 -> Check_Message_Service3D/3(CORRECTLY_ALTERED_INTO_MEMORY)
  Constant/1 -> Check_Message_Service3D/2(CONDITION_CORRECT)
  Check_Lenght_Message_Service3D/1(LENGTH_MESSAGE_CORRECT) -> Check_Message_Service3D/1(LENGTH_MESSAGE_CORRECT)
  REQ_DATA_NUMBER/1 -> Parameter_Valid_Service3D/1(REQ_DATA_NUMBER), Check_Lenght_Message_Service3D/1(REQ_DATA_NUMBER)
  Parameter_Valid_Service3D/1(SECURITY_ACCESS_ACCEPTED) -> Check_Message_Service3D/4(SECURITY_ACCESS_ACCEPTED)
  Parameter_Valid_Service3D/2(PARAMETER_VALID) -> Check_Message_Service3D/5(PARAMETER_VALID)

== system Renault_Library/Services ECU/Diag/RSA_DiagnosticManagement/DIAGNOSTIC_SANCTION_TOP/Service3E ==
  REQ_DATA_NUMBER : Inport
  DIAG_BUFFER_REQUEST : Inport  Port=2
  SESSION_ACTIVE : Inport  Port=3
  Trigger : TriggerPort
  Check_Lenght_Message_Service3E : Subsystem
  Check_Message_Service3E : Stateflow chart  TreatAsAtomicUnit=on
  SubFunction_In_Active_Session_Service3E : Subsystem
  SubFunction_Supported_Service3E : Subsystem
  SUPPRESS_POS_RES : Outport
  NEGATIVE_RES_CODE : Outport  Port=2
  SEND_RES_MESSAGE : Outport  Port=3
  wires:
  SubFunction_Supported_Service3E/1(SUBFUNCTION_SUPPORTED) -> Check_Message_Service3E/3(SUBFUNCTION_SUPPORTED)
  REQ_DATA_NUMBER/1 -> Check_Lenght_Message_Service3E/1(REQ_DATA_NUMBER)
  Check_Lenght_Message_Service3E/1(LENGTH_MESSAGE_CORRECT) -> Check_Message_Service3E/1(LENGTH_MESSAGE_CORRECT)
  SubFunction_Supported_Service3E/2(SUPPRESS_POS_RES) -> SUPPRESS_POS_RES/1
  SESSION_ACTIVE/1 -> SubFunction_In_Active_Session_Service3E/1(SESSION)
  SubFunction_In_Active_Session_Service3E/1(SUBFUNCTION_SUPPORTED_IN_ACTIVE_SESSION) -> Check_Message_Service3E/2(SUBFUNCTION_SUPPORTED_IN_ACTIVE_SESSION)
  DIAG_BUFFER_REQUEST/1 -> SubFunction_In_Active_Session_Service3E/2(DIAG_BUFFER_REQUEST), SubFunction_Supported_Service3E/1(DIAG_BUFFER_REQUEST)
  Check_Message_Service3E/2(SEND_RES_MESSAGE) -> SEND_RES_MESSAGE/1
  Check_Message_Service3E/1(NEGATIVE_RES_CODE) -> NEGATIVE_RES_CODE/1

== system Renault_Library/Services ECU/Diag/RSA_DiagnosticManagement/DIAGNOSTIC_SANCTION_TOP/Service87 ==
  REQ_DATA_NUMBER : Inport
  DIAG_BUFFER_REQUEST : Inport  Port=2
  SESSION_ACTIVE : Inport  Port=3
  Trigger : TriggerPort
  Check_Lenght_Message_Service87 : Subsystem
  Check_Message_Service87 : Stateflow chart  TreatAsAtomicUnit=on
  Constant : Constant  Value=true  OutDataTypeStr=boolean  SampleTime=-1
  Constant1 : Constant  Value=true  OutDataTypeStr=boolean  SampleTime=-1
  Parameter_Valid_Service87 : Subsystem
  SubFunction_In_Active_Session_Service87 : Subsystem
  SubFunction_Supported_Service87 : Subsystem
  NEGATIVE_RES_CODE : Outport
  SUPPRESS_POS_RES : Outport  Port=2
  SEND_RES_MESSAGE : Outport  Port=3
  wires:
  Parameter_Valid_Service87/1(PARAMETER_VALID) -> Check_Message_Service87/5(PARAMETER_VALID)
  Constant1/1 -> Check_Message_Service87/3(REQ_SEQUENCE_OK)
  Constant/1 -> Check_Message_Service87/2(CONDITION_CORRECT)
  Check_Message_Service87/2(SEND_RES_MESSAGE) -> SEND_RES_MESSAGE/1
  SubFunction_In_Active_Session_Service87/1(SUBFUNCTION_SUPPORTED_IN_ACTIVE_SESSION) -> Check_Message_Service87/4(SUBFUNCTION_SUPPORTED_IN_ACTIVE_SESSION)
  SubFunction_Supported_Service87/2(SUPPRESS_POS_RES) -> SUPPRESS_POS_RES/1
  DIAG_BUFFER_REQUEST/1 -> SubFunction_Supported_Service87/1(DIAG_BUFFER_REQUEST), SubFunction_In_Active_Session_Service87/2(DIAG_BUFFER_REQUEST), Parameter_Valid_Service87/1(DIAG_BUFFER_REQUEST)
  SubFunction_Supported_Service87/1(SUBFUNCTION_SUPPORTED) -> Check_Message_Service87/6(SUBFUNCTION_SUPPORTED)
  Check_Message_Service87/1(NEGATIVE_RES_CODE) -> NEGATIVE_RES_CODE/1
  Check_Lenght_Message_Service87/1(LENGTH_MESSAGE_CORRECT) -> Check_Message_Service87/1(LENGTH_MESSAGE_CORRECT)
  REQ_DATA_NUMBER/1 -> Check_Lenght_Message_Service87/1(REQ_DATA_NUMBER)
  SESSION_ACTIVE/1 -> SubFunction_In_Active_Session_Service87/1(SESSION)

== system Renault_Library/Services ECU/Diag/RSA_DiagnosticManagement/DIAGNOSTIC_SANCTION_TOP/SessionDiagnostic ==
  DIAG_BUFFER_REQUEST : Inport
  Diagnostic_Session_Control : Stateflow chart  TreatAsAtomicUnit=on
  Request_Of_Session : Stateflow chart  TreatAsAtomicUnit=on
  Tempo_Tester_Present : Stateflow chart  TreatAsAtomicUnit=on
  SESSION_ACTIVE : Outport  OutDataTypeStr=Enum: TYP_SESSION_ACTIVE
  wires:
  Request_Of_Session/1(Session) -> Diagnostic_Session_Control/2(Session)
  Diagnostic_Session_Control/1(SESSION_ACTIVE) -> SESSION_ACTIVE/1
  Tempo_Tester_Present/1(EV_TESTER_PRESENT) -> [EV_TESTER_PRESENT]
  [EV_TESTER_PRESENT] -> Diagnostic_Session_Control/1(EV_TESTER_PRESENT)
  DIAG_BUFFER_REQUEST/1 -> [DIAG_BUFFER_REQUEST]
  [DIAG_BUFFER_REQUEST] -> Request_Of_Session/1(DIAG_BUFFER_REQUEST)
  [DIAG_BUFFER_REQUEST] -> Tempo_Tester_Present/1(DIAG_BUFFER_REQUEST)

== system Renault_Library/Services ECU/Diag/RSA_DiagnosticManagement/DIAGNOSTIC_SEGMENTATION_TOP/Frame_Type ==
  DTOOL_TO_ECU : Inport
  BusSelector3 : BusSelector  OutputSignals=DTOOL_TO_ECU_PART1.DTOOL_TO_ECU_BYTE_0
  ShiftArithmetic : ArithShift  BitShiftNumber=4
  REQ_FRAME_TYPE : Outport  OutDataTypeStr=uint8
  wires:
  BusSelector3/1 -> ShiftArithmetic/1  "<DTOOL_TO_ECU_BYTE_0>"
  DTOOL_TO_ECU/1 -> BusSelector3/1  "DTOOL_TO_ECU"
  ShiftArithmetic/1 -> REQ_FRAME_TYPE/1

== system Renault_Library/Services ECU/Diag/RSA_DiagnosticManagement/DIAGNOSTIC_SEGMENTATION_TOP/Request_Frame ==
  PART1_DTOOL_TO_ECU : Inport
  PART2_DTOOL_TO_ECU : Inport  Port=2
  BitwiseOperator{,1..7} (8 alike) : simulink/Logic and Bit Operations/Bitwise Operator  logicop=AND  UseBitMask=on  BitMask=bin2dec('11111111')  BitMaskRealWorld=Stored Integer
  BusCreator : BusCreator
  BusCreator1 : BusCreator
  BusCreator2 : BusCreator  Inputs=2
  DataTypeConversion{,1..7} (8 alike) : DataTypeConversion  OutDataTypeStr=uint8  ConvertRealWorld=Stored Integer (SI)  RndMeth=Zero
  ShiftArithmetic{,1..5} (6 alike) : ArithShift  BitShiftNumber=8
  DTOOL_TO_ECU : Outport  OutDataTypeStr=uint8  PortDimensions=8
  wires:
  DataTypeConversion1/1 -> BusCreator1/2  "DTOOL_TO_ECU_BYTE_1"
  DataTypeConversion2/1 -> BusCreator1/3  "DTOOL_TO_ECU_BYTE_2"
  DataTypeConversion3/1 -> BusCreator1/4  "DTOOL_TO_ECU_BYTE_3"
  DataTypeConversion4/1 -> BusCreator/1  "DTOOL_TO_ECU_BYTE_4"
  DataTypeConversion5/1 -> BusCreator/2  "DTOOL_TO_ECU_BYTE_5"
  DataTypeConversion6/1 -> BusCreator/3  "DTOOL_TO_ECU_BYTE_6"
  BusCreator2/1 -> DTOOL_TO_ECU/1  "DTOOL_TO_ECU"
  DataTypeConversion/1 -> BusCreator1/1  "DTOOL_TO_ECU_BYTE_0"
  PART1_DTOOL_TO_ECU/1 -> ShiftArithmetic/1, BitwiseOperator3/1
  ShiftArithmetic/1 -> ShiftArithmetic1/1, BitwiseOperator2/1
  ShiftArithmetic1/1 -> ShiftArithmetic2/1, BitwiseOperator1/1
  ShiftArithmetic2/1 -> BitwiseOperator/1
  BitwiseOperator/1 -> DataTypeConversion/1
  BitwiseOperator1/1 -> DataTypeConversion1/1
  BitwiseOperator2/1 -> DataTypeConversion2/1
  BitwiseOperator3/1 -> DataTypeConversion3/1
  PART2_DTOOL_TO_ECU/1 -> ShiftArithmetic3/1, BitwiseOperator7/1
  ShiftArithmetic3/1 -> ShiftArithmetic4/1, BitwiseOperator6/1
  ShiftArithmetic4/1 -> ShiftArithmetic5/1, BitwiseOperator5/1
  ShiftArithmetic5/1 -> BitwiseOperator4/1
  BusCreator/1 -> BusCreator2/2  "DTOOL_TO_ECU_PART2"
  BusCreator1/1 -> BusCreator2/1  "DTOOL_TO_ECU_PART1"
  BitwiseOperator4/1 -> DataTypeConversion4/1
  BitwiseOperator5/1 -> DataTypeConversion5/1
  BitwiseOperator6/1 -> DataTypeConversion6/1
  BitwiseOperator7/1 -> DataTypeConversion7/1
  DataTypeConversion7/1 -> BusCreator/4  "DTOOL_TO_ECU_BYTE_7"

== system Renault_Library/Services ECU/Diag/RSA_DiagnosticManagement/DIAGNOSTIC_SEGMENTATION_TOP/Response_Frame ==
  ECU_TO_DTOOL : Inport  OutDataTypeStr=uint8  PortDimensions=8
  BusSelector1 : BusSelector  OutputSignals=ECU_TO_DTOOL_BYTE_0,ECU_TO_DTOOL_BYTE_1,ECU_TO_DTOOL_BYTE_2,ECU_TO_DTOOL_BYTE_3,ECU_TO_DTOOL_BYTE_4,ECU_TO_DTOOL_BYTE_5,ECU_TO_DTOOL_BYTE_6,ECU_TO_DTOOL_BYTE_7
  DataTypeConversion{1..8} (8 alike) : DataTypeConversion  OutDataTypeStr=uint32  ConvertRealWorld=Stored Integer (SI)  RndMeth=Zero
  ShiftArithmetic{,1..5} (6 alike) : ArithShift  BitShiftNumber=-8
  Sum{1..5,8} (6 alike) : Sum  OutDataTypeStr=Inherit: Inherit via back propagation  RndMeth=Zero  InputSameDT=on
  PART1_ECU_TO_DTOOL : Outport  OutDataTypeStr=uint32
  PART2_ECU_TO_DTOOL : Outport  Port=2  OutDataTypeStr=uint32
  wires:
  BusSelector1/1 -> DataTypeConversion7/1  "<ECU_TO_DTOOL_BYTE_0>"
  ShiftArithmetic/1 -> Sum8/1
  BusSelector1/2 -> DataTypeConversion1/1  "<ECU_TO_DTOOL_BYTE_1>"
  ShiftArithmetic1/1 -> Sum1/1
  BusSelector1/3 -> DataTypeConversion3/1  "<ECU_TO_DTOOL_BYTE_2>"
  Sum1/1 -> ShiftArithmetic2/1
  BusSelector1/4 -> DataTypeConversion2/1  "<ECU_TO_DTOOL_BYTE_3>"
  Sum2/1 -> PART1_ECU_TO_DTOOL/1
  BusSelector1/5 -> DataTypeConversion8/1  "<ECU_TO_DTOOL_BYTE_4>"
  ShiftArithmetic3/1 -> Sum3/1
  BusSelector1/6 -> DataTypeConversion4/1  "<ECU_TO_DTOOL_BYTE_5>"
  Sum3/1 -> ShiftArithmetic4/1
  ShiftArithmetic4/1 -> Sum4/1
  BusSelector1/7 -> DataTypeConversion6/1  "<ECU_TO_DTOOL_BYTE_6>"
  Sum4/1 -> ShiftArithmetic5/1
  BusSelector1/8 -> DataTypeConversion5/1  "<ECU_TO_DTOOL_BYTE_7>"
  ShiftArithmetic5/1 -> Sum5/1
  Sum5/1 -> PART2_ECU_TO_DTOOL/1
  DataTypeConversion8/1 -> ShiftArithmetic3/1
  DataTypeConversion4/1 -> Sum3/2
  DataTypeConversion6/1 -> Sum4/2
  DataTypeConversion2/1 -> Sum2/2
  DataTypeConversion3/1 -> Sum1/2
  DataTypeConversion1/1 -> Sum8/2
  DataTypeConversion7/1 -> ShiftArithmetic/1
  ECU_TO_DTOOL/1 -> BusSelector1/1  "ECU_TO_DTOOL"
  Sum8/1 -> ShiftArithmetic1/1
  ShiftArithmetic2/1 -> Sum2/1
  DataTypeConversion5/1 -> Sum5/2

== system Renault_Library/Services ECU/Diag/RSA_DiagnosticManagement_v02.00/DIAGNOSTIC_SANCTION_TOP/GeneralService ==
  SEG_STATE : Inport
  DIAG_BUFFER_REQUEST : Inport  Port=2
  SESSION_ACTIVE : Inport  Port=3
  REQ_DATA_NUMBER : Inport  Port=4
  General_Server_Response : Stateflow chart  TreatAsAtomicUnit=on
  Server_State : Subsystem
  Service_Supported : Subsystem
  Service_Supported_In_Active_Session : Subsystem
  NEGATIVE_RES_CODE : Outport
  NUMBER_SERVICE : Outport  Port=2  OutDataTypeStr=uint8
  SEND_RES_MESSAGE : Outport  Port=3  OutDataTypeStr=boolean
  REQUEST_RECEIVED : Outport  Port=4  OutDataTypeStr=boolean
  wires:
  General_Server_Response/1(NEGATIVE_RES_CODE) -> NEGATIVE_RES_CODE/1
  SEG_STATE/1 -> Server_State/1(SEG_STATE)
  DIAG_BUFFER_REQUEST/1 -> Service_Supported/1(DIAG_BUFFER_REQUEST), Service_Supported_In_Active_Session/1(DIAG_BUFFER_REQUEST), General_Server_Response/2(DIAG_BUFFER_REQUEST)
  Service_Supported/1(SERVICE_SUPPORTED) -> General_Server_Response/3(SERVICE_SUPPORTED)
  SESSION_ACTIVE/1 -> Service_Supported_In_Active_Session/2(SESSION_ACTIVE)
  Server_State/1(ECU_AVAILABLE) -> General_Server_Response/5(ECU_AVAILABLE)
  Service_Supported_In_Active_Session/1(SERVICE_SUPPORTED_IN_ACTIVE_SESSION) -> General_Server_Response/4(SERVICE_SUPPORTED_IN_ACTIVE_SESSION)
  General_Server_Response/2(NUMBER_SERVICE) -> NUMBER_SERVICE/1
  General_Server_Response/3(SEND_RES_MESSAGE) -> SEND_RES_MESSAGE/1
  General_Server_Response/4(REQUEST_RECEIVED) -> REQUEST_RECEIVED/1
  REQ_DATA_NUMBER/1 -> General_Server_Response/1(REQ_DATA_NUMBER)

== system Renault_Library/Services ECU/Diag/RSA_DiagnosticManagement_v02.00/DIAGNOSTIC_SANCTION_TOP/Service10 ==
  DIAG_BUFFER_REQUEST : Inport
  REQ_DATA_NUMBER : Inport  Port=2
  Trigger : TriggerPort
  Check_Lenght_Message_Service10 : Subsystem
  Check_Message_Service10 : Stateflow chart  TreatAsAtomicUnit=on
  Constant : Constant  Value=true  OutDataTypeStr=boolean  SampleTime=-1
  SubFunction_Supported_Service10 : Subsystem
  SUPPRESS_POS_RES : Outport
  NEGATIVE_RES_CODE : Outport  Port=2
  SEND_RES_MESSAGE : Outport  Port=3
  wires:
  REQ_DATA_NUMBER/1 -> Check_Lenght_Message_Service10/1(REQ_DATA_NUMBER)
  SubFunction_Supported_Service10/2(SUBFUNCTION_SUPPORTED) -> Check_Message_Service10/1(SUBFUNCTION_SUPPORTED)
  Check_Lenght_Message_Service10/1(LENGTH_MESSAGE_CORRECT) -> Check_Message_Service10/2(LENGTH_MESSAGE_CORRECT)
  DIAG_BUFFER_REQUEST/1 -> SubFunction_Supported_Service10/1(DIAG_BUFFER_REQUEST)
  SubFunction_Supported_Service10/1(SUPPRESS_POS_RES) -> SUPPRESS_POS_RES/1
  Check_Message_Service10/1(NEGATIVE_RES_CODE) -> NEGATIVE_RES_CODE/1
  Constant/1 -> Check_Message_Service10/3(CONDITION_CORRECT)
  Check_Message_Service10/2(SEND_RES_MESSAGE) -> SEND_RES_MESSAGE/1

== system Renault_Library/Services ECU/Diag/RSA_DiagnosticManagement_v02.00/DIAGNOSTIC_SANCTION_TOP/Service11 ==
  DIAG_BUFFER_REQUEST : Inport
  REQ_DATA_NUMBER : Inport  Port=2
  SESSION_ACTIVE : Inport  Port=3
  Trigger : TriggerPort
  Check_Lenght_Message_Service11 : Subsystem
  Check_Message_Service11 : Stateflow chart  TreatAsAtomicUnit=on
  Constant : Constant  Value=true  OutDataTypeStr=boolean  SampleTime=-1
  Constant1 : Constant  Value=true  OutDataTypeStr=boolean  SampleTime=-1
  SubFunction_In_Active_Session_Service11 : Subsystem
  SubFunction_Supported_Service11 : Subsystem
  SUPPRESS_POS_RES : Outport
  NEGATIVE_RES_CODE : Outport  Port=2
  SEND_RES_MESSAGE : Outport  Port=3
  wires:
  SESSION_ACTIVE/1 -> SubFunction_In_Active_Session_Service11/2(SESSION)
  Check_Message_Service11/1(NEGATIVE_RES_CODE) -> NEGATIVE_RES_CODE/1
  SubFunction_Supported_Service11/1(SUPPRESS_POS_RES) -> SUPPRESS_POS_RES/1
  Check_Lenght_Message_Service11/1(LENGTH_MESSAGE_CORRECT) -> Check_Message_Service11/3(LENGTH_MESSAGE_CORRECT)
  SubFunction_In_Active_Session_Service11/1(SUBFUNCTION_SUPPORTED_IN_ACTIVE_SESSION) -> Check_Message_Service11/2(SUBFUNCTION_SUPPORTED_IN_ACTIVE_SESSION)
  SubFunction_Supported_Service11/2(SUBFUNCTION_SUPPORTED) -> Check_Message_Service11/1(SUBFUNCTION_SUPPORTED)
  REQ_DATA_NUMBER/1 -> Check_Lenght_Message_Service11/1(REQ_DATA_NUMBER)
  DIAG_BUFFER_REQUEST/1 -> SubFunction_In_Active_Session_Service11/1(DIAG_BUFFER_REQUEST), SubFunction_Supported_Service11/1(DIAG_BUFFER_REQUEST)
  Check_Message_Service11/2(SEND_RES_MESSAGE) -> SEND_RES_MESSAGE/1
  Constant/1 -> Check_Message_Service11/4(CONDITION_CORRECT)
  Constant1/1 -> Check_Message_Service11/5(SECURITY_ACCESS_ACCEPTED)

== system Renault_Library/Services ECU/Diag/RSA_DiagnosticManagement_v02.00/DIAGNOSTIC_SANCTION_TOP/Service14 ==
  REQ_DATA_NUMBER : Inport
  SESSION_ACTIVE : Inport  Port=2
  DIAG_BUFFER_REQUEST : Inport  Port=3
  Trigger : TriggerPort
  Check_Lenght_Message_Service14 : Subsystem
  Check_Message_Service14 : Stateflow chart  TreatAsAtomicUnit=on
  Constant : Constant  Value=true  OutDataTypeStr=boolean  SampleTime=-1
  Parameter_Valid_Service14 : Subsystem
  NEGATIVE_RES_CODE : Outport
  SEND_RES_MESSAGE : Outport  Port=2
  wires:
  REQ_DATA_NUMBER/1 -> Check_Lenght_Message_Service14/1(REQ_DATA_NUMBER)
  Check_Lenght_Message_Service14/1(LENGTH_MESSAGE_CORRECT) -> Check_Message_Service14/1(LENGTH_MESSAGE_CORRECT)
  Check_Message_Service14/1(NEGATIVE_RES_CODE) -> NEGATIVE_RES_CODE/1
  Check_Message_Service14/2(SEND_RES_MESSAGE) -> SEND_RES_MESSAGE/1
  SESSION_ACTIVE/1 -> Parameter_Valid_Service14/2(SESSION_ACTIVE)
  Parameter_Valid_Service14/1(PARAMETER_VALID) -> Check_Message_Service14/3(PARAMETER_VALID)
  DIAG_BUFFER_REQUEST/1 -> Parameter_Valid_Service14/1(DIAG_BUFFER_REQUEST)
  Constant/1 -> Check_Message_Service14/2(CONDITION_CORRECT)
